simulink model "l1_ver106"  (Simulink 7.9)
settings: Solver=FixedStepDiscrete  SolverMode=SingleTasking  StartTime=0.0  StopTime=2048  RelTol=1e-3
undefined symbols (not defined in the file): sfp
libraries: xbsIndex_r4 x1795, casper_library_downconverter x124, xps_library x32, dspsigops x32, casper_library_misc x23, casper_library_flow_control x16, simulink x7
(part 3 of 16)

== system l1_ver106/converter28 ==
  u8_0 : Inport
  Concat : xbsIndex_r4/Concat  num_inputs=2  dbl_ovrd=off  block_type=concat
  Inverter1 : xbsIndex_r4/Inverter  en=off  latency=0  dbl_ovrd=off  block_type=inv
  Reinterpret : xbsIndex_r4/Reinterpret  force_arith_type=on  arith_type="Signed  (2's comp)"  force_bin_pt=on  bin_pt=7  block_type=reinterpret
  Slice : xbsIndex_r4/Slice  nbits=1  boolean_output=off  mode=Upper Bit Location + Width  bit1=0  base1=MSB of Input  bit0=0  base0=LSB of Input  dbl_ovrd=off  block_type=slice
  Slice1 : xbsIndex_r4/Slice  nbits=7  boolean_output=off  mode=Lower Bit Location + Width  bit1=0  base1=MSB of Input  bit0=0  base0=LSB of Input  dbl_ovrd=off  block_type=slice
  s8_7 : Outport
  wires:
  u8_0/1 -> Slice1/1, Slice/1
  Slice/1 -> Inverter1/1
  Concat/1 -> Reinterpret/1
  Inverter1/1 -> Concat/1
  Slice1/1 -> Concat/2
  Reinterpret/1 -> s8_7/1

== system l1_ver106/converter29 ==
  u8_0 : Inport
  Concat : xbsIndex_r4/Concat  num_inputs=2  dbl_ovrd=off  block_type=concat
  Inverter1 : xbsIndex_r4/Inverter  en=off  latency=0  dbl_ovrd=off  block_type=inv
  Reinterpret : xbsIndex_r4/Reinterpret  force_arith_type=on  arith_type="Signed  (2's comp)"  force_bin_pt=on  bin_pt=7  block_type=reinterpret
  Slice : xbsIndex_r4/Slice  nbits=1  boolean_output=off  mode=Upper Bit Location + Width  bit1=0  base1=MSB of Input  bit0=0  base0=LSB of Input  dbl_ovrd=off  block_type=slice
  Slice1 : xbsIndex_r4/Slice  nbits=7  boolean_output=off  mode=Lower Bit Location + Width  bit1=0  base1=MSB of Input  bit0=0  base0=LSB of Input  dbl_ovrd=off  block_type=slice
  s8_7 : Outport
  wires:
  Reinterpret/1 -> s8_7/1
  Slice1/1 -> Concat/2
  Inverter1/1 -> Concat/1
  Concat/1 -> Reinterpret/1
  Slice/1 -> Inverter1/1
  u8_0/1 -> Slice/1, Slice1/1

== system l1_ver106/converter3 ==
  u8_0 : Inport
  Concat : xbsIndex_r4/Concat  num_inputs=2  dbl_ovrd=off  block_type=concat
  Inverter1 : xbsIndex_r4/Inverter  en=off  latency=0  dbl_ovrd=off  block_type=inv
  Reinterpret : xbsIndex_r4/Reinterpret  force_arith_type=on  arith_type="Signed  (2's comp)"  force_bin_pt=on  bin_pt=7  block_type=reinterpret
  Slice : xbsIndex_r4/Slice  nbits=1  boolean_output=off  mode=Upper Bit Location + Width  bit1=0  base1=MSB of Input  bit0=0  base0=LSB of Input  dbl_ovrd=off  block_type=slice
  Slice1 : xbsIndex_r4/Slice  nbits=7  boolean_output=off  mode=Lower Bit Location + Width  bit1=0  base1=MSB of Input  bit0=0  base0=LSB of Input  dbl_ovrd=off  block_type=slice
  s8_7 : Outport
  wires:
  Reinterpret/1 -> s8_7/1
  Slice1/1 -> Concat/2
  Inverter1/1 -> Concat/1
  Concat/1 -> Reinterpret/1
  Slice/1 -> Inverter1/1
  u8_0/1 -> Slice/1, Slice1/1

== system l1_ver106/converter30 ==
  u8_0 : Inport
  Concat : xbsIndex_r4/Concat  num_inputs=2  dbl_ovrd=off  block_type=concat
  Inverter1 : xbsIndex_r4/Inverter  en=off  latency=0  dbl_ovrd=off  block_type=inv
  Reinterpret : xbsIndex_r4/Reinterpret  force_arith_type=on  arith_type="Signed  (2's comp)"  force_bin_pt=on  bin_pt=7  block_type=reinterpret
  Slice : xbsIndex_r4/Slice  nbits=1  boolean_output=off  mode=Upper Bit Location + Width  bit1=0  base1=MSB of Input  bit0=0  base0=LSB of Input  dbl_ovrd=off  block_type=slice
  Slice1 : xbsIndex_r4/Slice  nbits=7  boolean_output=off  mode=Lower Bit Location + Width  bit1=0  base1=MSB of Input  bit0=0  base0=LSB of Input  dbl_ovrd=off  block_type=slice
  s8_7 : Outport
  wires:
  u8_0/1 -> Slice1/1, Slice/1
  Slice/1 -> Inverter1/1
  Concat/1 -> Reinterpret/1
  Inverter1/1 -> Concat/1
  Slice1/1 -> Concat/2
  Reinterpret/1 -> s8_7/1

== system l1_ver106/converter31 ==
  u8_0 : Inport
  Concat : xbsIndex_r4/Concat  num_inputs=2  dbl_ovrd=off  block_type=concat
  Inverter1 : xbsIndex_r4/Inverter  en=off  latency=0  dbl_ovrd=off  block_type=inv
  Reinterpret : xbsIndex_r4/Reinterpret  force_arith_type=on  arith_type="Signed  (2's comp)"  force_bin_pt=on  bin_pt=7  block_type=reinterpret
  Slice : xbsIndex_r4/Slice  nbits=1  boolean_output=off  mode=Upper Bit Location + Width  bit1=0  base1=MSB of Input  bit0=0  base0=LSB of Input  dbl_ovrd=off  block_type=slice
  Slice1 : xbsIndex_r4/Slice  nbits=7  boolean_output=off  mode=Lower Bit Location + Width  bit1=0  base1=MSB of Input  bit0=0  base0=LSB of Input  dbl_ovrd=off  block_type=slice
  s8_7 : Outport
  wires:
  u8_0/1 -> Slice1/1, Slice/1
  Slice/1 -> Inverter1/1
  Concat/1 -> Reinterpret/1
  Inverter1/1 -> Concat/1
  Slice1/1 -> Concat/2
  Reinterpret/1 -> s8_7/1

== system l1_ver106/converter4 ==
  u8_0 : Inport
  Concat : xbsIndex_r4/Concat  num_inputs=2  dbl_ovrd=off  block_type=concat
  Inverter1 : xbsIndex_r4/Inverter  en=off  latency=0  dbl_ovrd=off  block_type=inv
  Reinterpret : xbsIndex_r4/Reinterpret  force_arith_type=on  arith_type="Signed  (2's comp)"  force_bin_pt=on  bin_pt=7  block_type=reinterpret
  Slice : xbsIndex_r4/Slice  nbits=1  boolean_output=off  mode=Upper Bit Location + Width  bit1=0  base1=MSB of Input  bit0=0  base0=LSB of Input  dbl_ovrd=off  block_type=slice
  Slice1 : xbsIndex_r4/Slice  nbits=7  boolean_output=off  mode=Lower Bit Location + Width  bit1=0  base1=MSB of Input  bit0=0  base0=LSB of Input  dbl_ovrd=off  block_type=slice
  s8_7 : Outport
  wires:
  u8_0/1 -> Slice1/1, Slice/1
  Slice/1 -> Inverter1/1
  Concat/1 -> Reinterpret/1
  Inverter1/1 -> Concat/1
  Slice1/1 -> Concat/2
  Reinterpret/1 -> s8_7/1

== system l1_ver106/converter5 ==
  u8_0 : Inport
  Concat : xbsIndex_r4/Concat  num_inputs=2  dbl_ovrd=off  block_type=concat
  Inverter1 : xbsIndex_r4/Inverter  en=off  latency=0  dbl_ovrd=off  block_type=inv
  Reinterpret : xbsIndex_r4/Reinterpret  force_arith_type=on  arith_type="Signed  (2's comp)"  force_bin_pt=on  bin_pt=7  block_type=reinterpret
  Slice : xbsIndex_r4/Slice  nbits=1  boolean_output=off  mode=Upper Bit Location + Width  bit1=0  base1=MSB of Input  bit0=0  base0=LSB of Input  dbl_ovrd=off  block_type=slice
  Slice1 : xbsIndex_r4/Slice  nbits=7  boolean_output=off  mode=Lower Bit Location + Width  bit1=0  base1=MSB of Input  bit0=0  base0=LSB of Input  dbl_ovrd=off  block_type=slice
  s8_7 : Outport
  wires:
  Reinterpret/1 -> s8_7/1
  Slice1/1 -> Concat/2
  Inverter1/1 -> Concat/1
  Concat/1 -> Reinterpret/1
  Slice/1 -> Inverter1/1
  u8_0/1 -> Slice/1, Slice1/1

== system l1_ver106/converter6 ==
  u8_0 : Inport
  Concat : xbsIndex_r4/Concat  num_inputs=2  dbl_ovrd=off  block_type=concat
  Inverter1 : xbsIndex_r4/Inverter  en=off  latency=0  dbl_ovrd=off  block_type=inv
  Reinterpret : xbsIndex_r4/Reinterpret  force_arith_type=on  arith_type="Signed  (2's comp)"  force_bin_pt=on  bin_pt=7  block_type=reinterpret
  Slice : xbsIndex_r4/Slice  nbits=1  boolean_output=off  mode=Upper Bit Location + Width  bit1=0  base1=MSB of Input  bit0=0  base0=LSB of Input  dbl_ovrd=off  block_type=slice
  Slice1 : xbsIndex_r4/Slice  nbits=7  boolean_output=off  mode=Lower Bit Location + Width  bit1=0  base1=MSB of Input  bit0=0  base0=LSB of Input  dbl_ovrd=off  block_type=slice
  s8_7 : Outport
  wires:
  u8_0/1 -> Slice1/1, Slice/1
  Slice/1 -> Inverter1/1
  Concat/1 -> Reinterpret/1
  Inverter1/1 -> Concat/1
  Slice1/1 -> Concat/2
  Reinterpret/1 -> s8_7/1

== system l1_ver106/converter7 ==
  u8_0 : Inport
  Concat : xbsIndex_r4/Concat  num_inputs=2  dbl_ovrd=off  block_type=concat
  Inverter1 : xbsIndex_r4/Inverter  en=off  latency=0  dbl_ovrd=off  block_type=inv
  Reinterpret : xbsIndex_r4/Reinterpret  force_arith_type=on  arith_type="Signed  (2's comp)"  force_bin_pt=on  bin_pt=7  block_type=reinterpret
  Slice : xbsIndex_r4/Slice  nbits=1  boolean_output=off  mode=Upper Bit Location + Width  bit1=0  base1=MSB of Input  bit0=0  base0=LSB of Input  dbl_ovrd=off  block_type=slice
  Slice1 : xbsIndex_r4/Slice  nbits=7  boolean_output=off  mode=Lower Bit Location + Width  bit1=0  base1=MSB of Input  bit0=0  base0=LSB of Input  dbl_ovrd=off  block_type=slice
  s8_7 : Outport
  wires:
  Reinterpret/1 -> s8_7/1
  Slice1/1 -> Concat/2
  Inverter1/1 -> Concat/1
  Concat/1 -> Reinterpret/1
  Slice/1 -> Inverter1/1
  u8_0/1 -> Slice/1, Slice1/1

== system l1_ver106/converter8 ==
  u8_0 : Inport
  Concat : xbsIndex_r4/Concat  num_inputs=2  dbl_ovrd=off  block_type=concat
  Inverter1 : xbsIndex_r4/Inverter  en=off  latency=0  dbl_ovrd=off  block_type=inv
  Reinterpret : xbsIndex_r4/Reinterpret  force_arith_type=on  arith_type="Signed  (2's comp)"  force_bin_pt=on  bin_pt=7  block_type=reinterpret
  Slice : xbsIndex_r4/Slice  nbits=1  boolean_output=off  mode=Upper Bit Location + Width  bit1=0  base1=MSB of Input  bit0=0  base0=LSB of Input  dbl_ovrd=off  block_type=slice
  Slice1 : xbsIndex_r4/Slice  nbits=7  boolean_output=off  mode=Lower Bit Location + Width  bit1=0  base1=MSB of Input  bit0=0  base0=LSB of Input  dbl_ovrd=off  block_type=slice
  s8_7 : Outport
  wires:
  u8_0/1 -> Slice1/1, Slice/1
  Slice/1 -> Inverter1/1
  Concat/1 -> Reinterpret/1
  Inverter1/1 -> Concat/1
  Slice1/1 -> Concat/2
  Reinterpret/1 -> s8_7/1

== system l1_ver106/converter9 ==
  u8_0 : Inport
  Concat : xbsIndex_r4/Concat  num_inputs=2  dbl_ovrd=off  block_type=concat
  Inverter1 : xbsIndex_r4/Inverter  en=off  latency=0  dbl_ovrd=off  block_type=inv
  Reinterpret : xbsIndex_r4/Reinterpret  force_arith_type=on  arith_type="Signed  (2's comp)"  force_bin_pt=on  bin_pt=7  block_type=reinterpret
  Slice : xbsIndex_r4/Slice  nbits=1  boolean_output=off  mode=Upper Bit Location + Width  bit1=0  base1=MSB of Input  bit0=0  base0=LSB of Input  dbl_ovrd=off  block_type=slice
  Slice1 : xbsIndex_r4/Slice  nbits=7  boolean_output=off  mode=Lower Bit Location + Width  bit1=0  base1=MSB of Input  bit0=0  base0=LSB of Input  dbl_ovrd=off  block_type=slice
  s8_7 : Outport
  wires:
  Reinterpret/1 -> s8_7/1
  Slice1/1 -> Concat/2
  Inverter1/1 -> Concat/1
  Concat/1 -> Reinterpret/1
  Slice/1 -> Inverter1/1
  u8_0/1 -> Slice/1, Slice1/1

== system l1_ver106/dec_fir1 ==
  sync_in : Inport
  real1 : Inport  Port=2
  imag1 : Inport  Port=3
  real2 : Inport  Port=4
  imag2 : Inport  Port=5
  real3 : Inport  Port=6
  imag3 : Inport  Port=7
  real4 : Inport  Port=8
  imag4 : Inport  Port=9
  real5 : Inport  Port=10
  imag5 : Inport  Port=11
  real6 : Inport  Port=12
  imag6 : Inport  Port=13
  real7 : Inport  Port=14
  imag7 : Inport  Port=15
  real8 : Inport  Port=16
  imag8 : Inport  Port=17
  real9 : Inport  Port=18
  imag9 : Inport  Port=19
  real10 : Inport  Port=20
  imag10 : Inport  Port=21
  real11 : Inport  Port=22
  imag11 : Inport  Port=23
  real12 : Inport  Port=24
  imag12 : Inport  Port=25
  real13 : Inport  Port=26
  imag13 : Inport  Port=27
  real14 : Inport  Port=28
  imag14 : Inport  Port=29
  real15 : Inport  Port=30
  imag15 : Inport  Port=31
  real16 : Inport  Port=32
  imag16 : Inport  Port=33
  convert1 : xbsIndex_r4/Convert  gui_display_data_type=Fixed-point  arith_type="Signed  (2's comp)"  n_bits=32  bin_pt=31  float_type=Single  exp_bits=8  fraction_bits=24  quantization="Round  (unbiased: +/- Inf)"  overflow=Wrap  en=off  latency=2  dbl_ovrd=off  pipeline=off  block_type=convert
  convert2 : xbsIndex_r4/Convert  gui_display_data_type=Fixed-point  arith_type="Signed  (2's comp)"  n_bits=32  bin_pt=31  float_type=Single  exp_bits=8  fraction_bits=24  quantization="Round  (unbiased: +/- Inf)"  overflow=Wrap  en=off  latency=2  dbl_ovrd=off  pipeline=off  block_type=convert
  delay : xbsIndex_r4/Delay  rst=off  infoeditControl=Selection of Reset will increase slice count due to use of real FFs and instead of SRLs  en=off  latency=9  dbl_ovrd=off  reg_retiming=off  block_type=delay
  fir_dbl_col1 : Subsystem  AncestorBlock=casper_library_downconverter/fir_dbl_col  mask(fir_dbl_col){n_inputs=16, coeff=[0.23734     0.22808     0.21853     0.20905     0.19939     0.18961     0.17952     0.16949      0.1593     0.14934     0.13944     0.12979     0.12023     0.11094     0.10175     0.53424], add_latency=1, mult_latency=2, coeff_bit_width=25, coeff_bin_pt=24, first_stage_hdl=on, adder_imp=DSP48}
      mask prompts: n_inputs "Inputs"; coeff "Coefficients"; add_latency "Add latency"; mult_latency "Mult Latency"; coeff_bit_width "Coefficient bit width"; coeff_bin_pt "Coefficient binary point"; first_stage_hdl "Implement first stage of adder trees on output as behavioural HDL"; adder_imp "Adder implementation"
      mask init:
      | fir_dbl_col_init(gcb, ...
      |     'n_inputs', n_inputs, ...
      |     'coeff', coeff, ...
      |     'mult_latency', mult_latency, ...
      |     'add_latency', add_latency, ...
      |     'coeff_bit_width', coeff_bit_width, ...
      |     'coeff_bin_pt', coeff_bin_pt,  ...
      |     'first_stage_hdl', first_stage_hdl, ...
      |     'adder_imp', adder_imp);
      mask Help: eval('xlWeb(''http://casper.berkeley.edu/wiki/Fir_dbl_col'')')
      mask StyleString: edit,edit,edit,edit,edit,edit,checkbox,popup(Behavioral|Fabric|DSP48)
  fir_dbl_col2 : Subsystem  AncestorBlock=casper_library_downconverter/fir_dbl_col  mask(fir_dbl_col){n_inputs=16, coeff=[0.32081     0.32018     0.31891     0.31676     0.31411     0.31084     0.30683     0.30225     0.29702     0.29121     0.28491     0.27801     0.27074     0.26309     0.25434     0.24631], add_latency=1, mult_latency=2, coeff_bit_width=25, coeff_bin_pt=24, first_stage_hdl=on, adder_imp=DSP48}
      mask prompts: n_inputs "Inputs"; coeff "Coefficients"; add_latency "Add latency"; mult_latency "Mult Latency"; coeff_bit_width "Coefficient bit width"; coeff_bin_pt "Coefficient binary point"; first_stage_hdl "Implement first stage of adder trees on output as behavioural HDL"; adder_imp "Adder implementation"
      mask init: same as l1_ver106/dec_fir1/fir_dbl_col1
      mask Help: eval('xlWeb(''http://casper.berkeley.edu/wiki/Fir_dbl_col'')')
      mask StyleString: edit,edit,edit,edit,edit,edit,checkbox,popup(Behavioral|Fabric|DSP48)
  imag_sum : Subsystem  AncestorBlock=casper_library_misc/adder_tree  mask(adder_tree){n_inputs=2, latency=1, adder_imp=DSP48, first_stage_hdl=off}
      mask prompts: n_inputs "Number of Inputs"; latency "Add Latency"; adder_imp "Adder implementation"; first_stage_hdl "Use behavioural HDL for first stage of adders"
      mask help:
      | Sums all inputs using a tree of adds and delays.
      |
      | Number of inputs: The number of inputs to the block to be summed
      | Add latency: The number of clock cycles to perform one addition (affects timing)..
      | Adder implementation: choice of fabric or DSP48E core, or behavioral HDL
      | Use behavioural HDL for first stage of adders: Causes the first stage of adders to be absorbed into DSP blocks if multipliers precede them (Virtex 5). Overrides Adder implementation
      mask init:
      | adder_tree_init(gcb, ...
      |     'n_inputs', n_inputs, ...
      |     'latency', latency, ...
      |     'adder_imp', adder_imp, ...
      |     'first_stage_hdl', first_stage_hdl);
      settings: AttributesFormatString=latency 1
      mask Help: eval('xlWeb(''http://casper.berkeley.edu/wiki/Adder_tree'')')
      mask StyleString: edit,edit,popup(Behavioral|Fabric|DSP48),checkbox
  real_sum : Subsystem  AncestorBlock=casper_library_misc/adder_tree  mask(adder_tree){n_inputs=2, latency=1, adder_imp=DSP48, first_stage_hdl=off}
      mask prompts: n_inputs "Number of Inputs"; latency "Add Latency"; adder_imp "Adder implementation"; first_stage_hdl "Use behavioural HDL for first stage of adders"
      mask help:
      | Sums all inputs using a tree of adds and delays.
      |
      | Number of inputs: The number of inputs to the block to be summed
      | Add latency: The number of clock cycles to perform one addition (affects timing)..
      | Adder implementation: choice of fabric or DSP48E core, or behavioral HDL
      | Use behavioural HDL for first stage of adders: Causes the first stage of adders to be absorbed into DSP blocks if multipliers precede them (Virtex 5). Overrides Adder implementation
      mask init:
      | adder_tree_init(gcb, ...
      |     'n_inputs', n_inputs, ...
      |     'latency', latency, ...
      |     'adder_imp', adder_imp, ...
      |     'first_stage_hdl', first_stage_hdl);
      settings: AttributesFormatString=latency 1
      mask Help: eval('xlWeb(''http://casper.berkeley.edu/wiki/Adder_tree'')')
      mask StyleString: edit,edit,popup(Behavioral|Fabric|DSP48),checkbox
  ri_to_c : casper_library_misc/ri_to_c
  shift1 : xbsIndex_r4/Shift  shift_dir=Left  shift_bits=1  en=off  latency=0  precision=Full  arith_type="Signed  (2's comp)"  n_bits=16  bin_pt=14  quantization=Truncate  overflow=Wrap  dbl_ovrd=off  block_type=shift
  shift2 : xbsIndex_r4/Shift  shift_dir=Left  shift_bits=1  en=off  latency=0  precision=Full  arith_type="Signed  (2's comp)"  n_bits=16  bin_pt=14  quantization=Truncate  overflow=Wrap  dbl_ovrd=off  block_type=shift
  sync_out : Outport
  dout : Outport  Port=2
  wires:
  real1/1 -> fir_dbl_col1/1(real1)
  imag1/1 -> fir_dbl_col1/2(imag1)
  real2/1 -> fir_dbl_col1/3(real2)
  imag2/1 -> fir_dbl_col1/4(imag2)
  real3/1 -> fir_dbl_col1/5(real3)
  imag3/1 -> fir_dbl_col1/6(imag3)
  real4/1 -> fir_dbl_col1/7(real4)
  imag4/1 -> fir_dbl_col1/8(imag4)
  real5/1 -> fir_dbl_col1/9(real5)
  imag5/1 -> fir_dbl_col1/10(imag5)
  real6/1 -> fir_dbl_col1/11(real6)
  imag6/1 -> fir_dbl_col1/12(imag6)
  real7/1 -> fir_dbl_col1/13(real7)
  imag7/1 -> fir_dbl_col1/14(imag7)
  real8/1 -> fir_dbl_col1/15(real8)
  imag8/1 -> fir_dbl_col1/16(imag8)
  real9/1 -> fir_dbl_col1/17(real9)
  imag9/1 -> fir_dbl_col1/18(imag9)
  real10/1 -> fir_dbl_col1/19(real10)
  imag10/1 -> fir_dbl_col1/20(imag10)
  real11/1 -> fir_dbl_col1/21(real11)
  imag11/1 -> fir_dbl_col1/22(imag11)
  real12/1 -> fir_dbl_col1/23(real12)
  imag12/1 -> fir_dbl_col1/24(imag12)
  real13/1 -> fir_dbl_col1/25(real13)
  imag13/1 -> fir_dbl_col1/26(imag13)
  real14/1 -> fir_dbl_col1/27(real14)
  imag14/1 -> fir_dbl_col1/28(imag14)
  real15/1 -> fir_dbl_col1/29(real15)
  imag15/1 -> fir_dbl_col1/30(imag15)
  real16/1 -> fir_dbl_col1/31(real16)
  imag16/1 -> fir_dbl_col1/32(imag16)
  fir_dbl_col1/65(real_sum) -> real_sum/2(din1)
  fir_dbl_col1/66(imag_sum) -> imag_sum/2(din1)
  fir_dbl_col1/1(real_out1) -> fir_dbl_col2/1(real1)
  fir_dbl_col1/2(imag_out1) -> fir_dbl_col2/2(imag1)
  fir_dbl_col1/3(real_out2) -> fir_dbl_col2/3(real2)
  fir_dbl_col1/4(imag_out2) -> fir_dbl_col2/4(imag2)
  fir_dbl_col1/5(real_out3) -> fir_dbl_col2/5(real3)
  fir_dbl_col1/6(imag_out3) -> fir_dbl_col2/6(imag3)
  fir_dbl_col1/7(real_out4) -> fir_dbl_col2/7(real4)
  fir_dbl_col1/8(imag_out4) -> fir_dbl_col2/8(imag4)
  fir_dbl_col1/9(real_out5) -> fir_dbl_col2/9(real5)
  fir_dbl_col1/10(imag_out5) -> fir_dbl_col2/10(imag5)
  fir_dbl_col1/11(real_out6) -> fir_dbl_col2/11(real6)
  fir_dbl_col1/12(imag_out6) -> fir_dbl_col2/12(imag6)
  fir_dbl_col1/13(real_out7) -> fir_dbl_col2/13(real7)
  fir_dbl_col1/14(imag_out7) -> fir_dbl_col2/14(imag7)
  fir_dbl_col1/15(real_out8) -> fir_dbl_col2/15(real8)
  fir_dbl_col1/16(imag_out8) -> fir_dbl_col2/16(imag8)
  fir_dbl_col1/17(real_out9) -> fir_dbl_col2/17(real9)
  fir_dbl_col1/18(imag_out9) -> fir_dbl_col2/18(imag9)
  fir_dbl_col1/19(real_out10) -> fir_dbl_col2/19(real10)
  fir_dbl_col1/20(imag_out10) -> fir_dbl_col2/20(imag10)
  fir_dbl_col1/21(real_out11) -> fir_dbl_col2/21(real11)
  fir_dbl_col1/22(imag_out11) -> fir_dbl_col2/22(imag11)
  fir_dbl_col1/23(real_out12) -> fir_dbl_col2/23(real12)
  fir_dbl_col1/24(imag_out12) -> fir_dbl_col2/24(imag12)
  fir_dbl_col1/25(real_out13) -> fir_dbl_col2/25(real13)
  fir_dbl_col1/26(imag_out13) -> fir_dbl_col2/26(imag13)
  fir_dbl_col1/27(real_out14) -> fir_dbl_col2/27(real14)
  fir_dbl_col1/28(imag_out14) -> fir_dbl_col2/28(imag14)
  fir_dbl_col1/29(real_out15) -> fir_dbl_col2/29(real15)
  fir_dbl_col1/30(imag_out15) -> fir_dbl_col2/30(imag15)
  fir_dbl_col1/31(real_out16) -> fir_dbl_col2/31(real16)
  fir_dbl_col1/32(imag_out16) -> fir_dbl_col2/32(imag16)
  fir_dbl_col2/65(real_sum) -> real_sum/3(din2)
  fir_dbl_col2/66(imag_sum) -> imag_sum/3(din2)
  real_sum/2(dout) -> shift1/1
  imag_sum/2(dout) -> shift2/1
  shift1/1 -> convert1/1
  shift2/1 -> convert2/1
  convert1/1 -> ri_to_c/1
  convert2/1 -> ri_to_c/2
  ri_to_c/1 -> dout/1
  sync_in/1 -> delay/1
  delay/1 -> real_sum/1(sync), imag_sum/1(sync)
  real_sum/1(sync_out) -> sync_out/1
  fir_dbl_col2/33(real_back_out1) -> fir_dbl_col1/33(real_back1)
  fir_dbl_col2/34(imag_back_out1) -> fir_dbl_col1/34(imag_back1)
  fir_dbl_col2/35(real_back_out2) -> fir_dbl_col1/35(real_back2)
  fir_dbl_col2/36(imag_back_out2) -> fir_dbl_col1/36(imag_back2)
  fir_dbl_col2/37(real_back_out3) -> fir_dbl_col1/37(real_back3)
  fir_dbl_col2/38(imag_back_out3) -> fir_dbl_col1/38(imag_back3)
  fir_dbl_col2/39(real_back_out4) -> fir_dbl_col1/39(real_back4)
  fir_dbl_col2/40(imag_back_out4) -> fir_dbl_col1/40(imag_back4)
  fir_dbl_col2/41(real_back_out5) -> fir_dbl_col1/41(real_back5)
  fir_dbl_col2/42(imag_back_out5) -> fir_dbl_col1/42(imag_back5)
  fir_dbl_col2/43(real_back_out6) -> fir_dbl_col1/43(real_back6)
  fir_dbl_col2/44(imag_back_out6) -> fir_dbl_col1/44(imag_back6)
  fir_dbl_col2/45(real_back_out7) -> fir_dbl_col1/45(real_back7)
  fir_dbl_col2/46(imag_back_out7) -> fir_dbl_col1/46(imag_back7)
  fir_dbl_col2/47(real_back_out8) -> fir_dbl_col1/47(real_back8)
  fir_dbl_col2/48(imag_back_out8) -> fir_dbl_col1/48(imag_back8)
  fir_dbl_col2/49(real_back_out9) -> fir_dbl_col1/49(real_back9)
  fir_dbl_col2/50(imag_back_out9) -> fir_dbl_col1/50(imag_back9)
  fir_dbl_col2/51(real_back_out10) -> fir_dbl_col1/51(real_back10)
  fir_dbl_col2/52(imag_back_out10) -> fir_dbl_col1/52(imag_back10)
  fir_dbl_col2/53(real_back_out11) -> fir_dbl_col1/53(real_back11)
  fir_dbl_col2/54(imag_back_out11) -> fir_dbl_col1/54(imag_back11)
  fir_dbl_col2/55(real_back_out12) -> fir_dbl_col1/55(real_back12)
  fir_dbl_col2/56(imag_back_out12) -> fir_dbl_col1/56(imag_back12)
  fir_dbl_col2/57(real_back_out13) -> fir_dbl_col1/57(real_back13)
  fir_dbl_col2/58(imag_back_out13) -> fir_dbl_col1/58(imag_back13)
  fir_dbl_col2/59(real_back_out14) -> fir_dbl_col1/59(real_back14)
  fir_dbl_col2/60(imag_back_out14) -> fir_dbl_col1/60(imag_back14)
  fir_dbl_col2/61(real_back_out15) -> fir_dbl_col1/61(real_back15)
  fir_dbl_col2/62(imag_back_out15) -> fir_dbl_col1/62(imag_back15)
  fir_dbl_col2/63(real_back_out16) -> fir_dbl_col1/63(real_back16)
  fir_dbl_col2/64(imag_back_out16) -> fir_dbl_col1/64(imag_back16)
  fir_dbl_col2/1(real_out1) -> fir_dbl_col2/33(real_back1)
  fir_dbl_col2/2(imag_out1) -> fir_dbl_col2/34(imag_back1)
  fir_dbl_col2/3(real_out2) -> fir_dbl_col2/35(real_back2)
  fir_dbl_col2/4(imag_out2) -> fir_dbl_col2/36(imag_back2)
  fir_dbl_col2/5(real_out3) -> fir_dbl_col2/37(real_back3)
  fir_dbl_col2/6(imag_out3) -> fir_dbl_col2/38(imag_back3)
  fir_dbl_col2/7(real_out4) -> fir_dbl_col2/39(real_back4)
  fir_dbl_col2/8(imag_out4) -> fir_dbl_col2/40(imag_back4)
  fir_dbl_col2/9(real_out5) -> fir_dbl_col2/41(real_back5)
  fir_dbl_col2/10(imag_out5) -> fir_dbl_col2/42(imag_back5)
  fir_dbl_col2/11(real_out6) -> fir_dbl_col2/43(real_back6)
  fir_dbl_col2/12(imag_out6) -> fir_dbl_col2/44(imag_back6)
  fir_dbl_col2/13(real_out7) -> fir_dbl_col2/45(real_back7)
  fir_dbl_col2/14(imag_out7) -> fir_dbl_col2/46(imag_back7)
  fir_dbl_col2/15(real_out8) -> fir_dbl_col2/47(real_back8)
  fir_dbl_col2/16(imag_out8) -> fir_dbl_col2/48(imag_back8)
  fir_dbl_col2/17(real_out9) -> fir_dbl_col2/49(real_back9)
  fir_dbl_col2/18(imag_out9) -> fir_dbl_col2/50(imag_back9)
  fir_dbl_col2/19(real_out10) -> fir_dbl_col2/51(real_back10)
  fir_dbl_col2/20(imag_out10) -> fir_dbl_col2/52(imag_back10)
  fir_dbl_col2/21(real_out11) -> fir_dbl_col2/53(real_back11)
  fir_dbl_col2/22(imag_out11) -> fir_dbl_col2/54(imag_back11)
  fir_dbl_col2/23(real_out12) -> fir_dbl_col2/55(real_back12)
  fir_dbl_col2/24(imag_out12) -> fir_dbl_col2/56(imag_back12)
  fir_dbl_col2/25(real_out13) -> fir_dbl_col2/57(real_back13)
  fir_dbl_col2/26(imag_out13) -> fir_dbl_col2/58(imag_back13)
  fir_dbl_col2/27(real_out14) -> fir_dbl_col2/59(real_back14)
  fir_dbl_col2/28(imag_out14) -> fir_dbl_col2/60(imag_back14)
  fir_dbl_col2/29(real_out15) -> fir_dbl_col2/61(real_back15)
  fir_dbl_col2/30(imag_out15) -> fir_dbl_col2/62(imag_back15)
  fir_dbl_col2/31(real_out16) -> fir_dbl_col2/63(real_back16)
  fir_dbl_col2/32(imag_out16) -> fir_dbl_col2/64(imag_back16)

== system l1_ver106/dec_fir3 ==
  sync_in : Inport
  real1 : Inport  Port=2
  imag1 : Inport  Port=3
  real2 : Inport  Port=4
  imag2 : Inport  Port=5
  real3 : Inport  Port=6
  imag3 : Inport  Port=7
  real4 : Inport  Port=8
  imag4 : Inport  Port=9
  real5 : Inport  Port=10
  imag5 : Inport  Port=11
  real6 : Inport  Port=12
  imag6 : Inport  Port=13
  real7 : Inport  Port=14
  imag7 : Inport  Port=15
  real8 : Inport  Port=16
  imag8 : Inport  Port=17
  real9 : Inport  Port=18
  imag9 : Inport  Port=19
  real10 : Inport  Port=20
  imag10 : Inport  Port=21
  real11 : Inport  Port=22
  imag11 : Inport  Port=23
  real12 : Inport  Port=24
  imag12 : Inport  Port=25
  real13 : Inport  Port=26
  imag13 : Inport  Port=27
  real14 : Inport  Port=28
  imag14 : Inport  Port=29
  real15 : Inport  Port=30
  imag15 : Inport  Port=31
  real16 : Inport  Port=32
  imag16 : Inport  Port=33
  convert1 : xbsIndex_r4/Convert  gui_display_data_type=Fixed-point  arith_type="Signed  (2's comp)"  n_bits=32  bin_pt=31  float_type=Single  exp_bits=8  fraction_bits=24  quantization="Round  (unbiased: +/- Inf)"  overflow=Wrap  en=off  latency=2  dbl_ovrd=off  pipeline=off  block_type=convert
  convert2 : xbsIndex_r4/Convert  gui_display_data_type=Fixed-point  arith_type="Signed  (2's comp)"  n_bits=32  bin_pt=31  float_type=Single  exp_bits=8  fraction_bits=24  quantization="Round  (unbiased: +/- Inf)"  overflow=Wrap  en=off  latency=2  dbl_ovrd=off  pipeline=off  block_type=convert
  delay : xbsIndex_r4/Delay  rst=off  infoeditControl=Selection of Reset will increase slice count due to use of real FFs and instead of SRLs  en=off  latency=9  dbl_ovrd=off  reg_retiming=off  block_type=delay
  fir_dbl_col1 : Subsystem  AncestorBlock=casper_library_downconverter/fir_dbl_col  mask(fir_dbl_col){n_inputs=16, coeff=[0.23734     0.22808     0.21853     0.20905     0.19939     0.18961     0.17952     0.16949      0.1593     0.14934     0.13944     0.12979     0.12023     0.11094     0.10175     0.53424], add_latency=1, mult_latency=2, coeff_bit_width=25, coeff_bin_pt=24, first_stage_hdl=on, adder_imp=DSP48}
      mask prompts: n_inputs "Inputs"; coeff "Coefficients"; add_latency "Add latency"; mult_latency "Mult Latency"; coeff_bit_width "Coefficient bit width"; coeff_bin_pt "Coefficient binary point"; first_stage_hdl "Implement first stage of adder trees on output as behavioural HDL"; adder_imp "Adder implementation"
      mask init: same as l1_ver106/dec_fir1/fir_dbl_col1
      mask Help: eval('xlWeb(''http://casper.berkeley.edu/wiki/Fir_dbl_col'')')
      mask StyleString: edit,edit,edit,edit,edit,edit,checkbox,popup(Behavioral|Fabric|DSP48)
  fir_dbl_col2 : Subsystem  AncestorBlock=casper_library_downconverter/fir_dbl_col  mask(fir_dbl_col){n_inputs=16, coeff=[0.32081     0.32018     0.31891     0.31676     0.31411     0.31084     0.30683     0.30225     0.29702     0.29121     0.28491     0.27801     0.27074     0.26309     0.25434     0.24631], add_latency=1, mult_latency=2, coeff_bit_width=25, coeff_bin_pt=24, first_stage_hdl=on, adder_imp=DSP48}
      mask prompts: n_inputs "Inputs"; coeff "Coefficients"; add_latency "Add latency"; mult_latency "Mult Latency"; coeff_bit_width "Coefficient bit width"; coeff_bin_pt "Coefficient binary point"; first_stage_hdl "Implement first stage of adder trees on output as behavioural HDL"; adder_imp "Adder implementation"
      mask init: same as l1_ver106/dec_fir1/fir_dbl_col1
      mask Help: eval('xlWeb(''http://casper.berkeley.edu/wiki/Fir_dbl_col'')')
      mask StyleString: edit,edit,edit,edit,edit,edit,checkbox,popup(Behavioral|Fabric|DSP48)
  imag_sum : Subsystem  AncestorBlock=casper_library_misc/adder_tree  mask(adder_tree){n_inputs=2, latency=1, adder_imp=DSP48, first_stage_hdl=off}
      mask prompts: n_inputs "Number of Inputs"; latency "Add Latency"; adder_imp "Adder implementation"; first_stage_hdl "Use behavioural HDL for first stage of adders"
      mask help:
      | Sums all inputs using a tree of adds and delays.
      |
      | Number of inputs: The number of inputs to the block to be summed
      | Add latency: The number of clock cycles to perform one addition (affects timing)..
      | Adder implementation: choice of fabric or DSP48E core, or behavioral HDL
      | Use behavioural HDL for first stage of adders: Causes the first stage of adders to be absorbed into DSP blocks if multipliers precede them (Virtex 5). Overrides Adder implementation
      mask init:
      | adder_tree_init(gcb, ...
      |     'n_inputs', n_inputs, ...
      |     'latency', latency, ...
      |     'adder_imp', adder_imp, ...
      |     'first_stage_hdl', first_stage_hdl);
      settings: AttributesFormatString=latency 1
      mask Help: eval('xlWeb(''http://casper.berkeley.edu/wiki/Adder_tree'')')
      mask StyleString: edit,edit,popup(Behavioral|Fabric|DSP48),checkbox
  real_sum : Subsystem  AncestorBlock=casper_library_misc/adder_tree  mask(adder_tree){n_inputs=2, latency=1, adder_imp=DSP48, first_stage_hdl=off}
      mask prompts: n_inputs "Number of Inputs"; latency "Add Latency"; adder_imp "Adder implementation"; first_stage_hdl "Use behavioural HDL for first stage of adders"
      mask help:
      | Sums all inputs using a tree of adds and delays.
      |
      | Number of inputs: The number of inputs to the block to be summed
      | Add latency: The number of clock cycles to perform one addition (affects timing)..
      | Adder implementation: choice of fabric or DSP48E core, or behavioral HDL
      | Use behavioural HDL for first stage of adders: Causes the first stage of adders to be absorbed into DSP blocks if multipliers precede them (Virtex 5). Overrides Adder implementation
      mask init:
      | adder_tree_init(gcb, ...
      |     'n_inputs', n_inputs, ...
      |     'latency', latency, ...
      |     'adder_imp', adder_imp, ...
      |     'first_stage_hdl', first_stage_hdl);
      settings: AttributesFormatString=latency 1
      mask Help: eval('xlWeb(''http://casper.berkeley.edu/wiki/Adder_tree'')')
      mask StyleString: edit,edit,popup(Behavioral|Fabric|DSP48),checkbox
  ri_to_c : casper_library_misc/ri_to_c
  shift1 : xbsIndex_r4/Shift  shift_dir=Left  shift_bits=1  en=off  latency=0  precision=Full  arith_type="Signed  (2's comp)"  n_bits=16  bin_pt=14  quantization=Truncate  overflow=Wrap  dbl_ovrd=off  block_type=shift
  shift2 : xbsIndex_r4/Shift  shift_dir=Left  shift_bits=1  en=off  latency=0  precision=Full  arith_type="Signed  (2's comp)"  n_bits=16  bin_pt=14  quantization=Truncate  overflow=Wrap  dbl_ovrd=off  block_type=shift
  sync_out : Outport
  dout : Outport  Port=2
  wires:
  real1/1 -> fir_dbl_col1/1(real1)
  imag1/1 -> fir_dbl_col1/2(imag1)
  real2/1 -> fir_dbl_col1/3(real2)
  imag2/1 -> fir_dbl_col1/4(imag2)
  real3/1 -> fir_dbl_col1/5(real3)
  imag3/1 -> fir_dbl_col1/6(imag3)
  real4/1 -> fir_dbl_col1/7(real4)
  imag4/1 -> fir_dbl_col1/8(imag4)
  real5/1 -> fir_dbl_col1/9(real5)
  imag5/1 -> fir_dbl_col1/10(imag5)
  real6/1 -> fir_dbl_col1/11(real6)
  imag6/1 -> fir_dbl_col1/12(imag6)
  real7/1 -> fir_dbl_col1/13(real7)
  imag7/1 -> fir_dbl_col1/14(imag7)
  real8/1 -> fir_dbl_col1/15(real8)
  imag8/1 -> fir_dbl_col1/16(imag8)
  real9/1 -> fir_dbl_col1/17(real9)
  imag9/1 -> fir_dbl_col1/18(imag9)
  real10/1 -> fir_dbl_col1/19(real10)
  imag10/1 -> fir_dbl_col1/20(imag10)
  real11/1 -> fir_dbl_col1/21(real11)
  imag11/1 -> fir_dbl_col1/22(imag11)
  real12/1 -> fir_dbl_col1/23(real12)
  imag12/1 -> fir_dbl_col1/24(imag12)
  real13/1 -> fir_dbl_col1/25(real13)
  imag13/1 -> fir_dbl_col1/26(imag13)
  real14/1 -> fir_dbl_col1/27(real14)
  imag14/1 -> fir_dbl_col1/28(imag14)
  real15/1 -> fir_dbl_col1/29(real15)
  imag15/1 -> fir_dbl_col1/30(imag15)
  real16/1 -> fir_dbl_col1/31(real16)
  imag16/1 -> fir_dbl_col1/32(imag16)
  fir_dbl_col1/65(real_sum) -> real_sum/2(din1)
  fir_dbl_col1/66(imag_sum) -> imag_sum/2(din1)
  fir_dbl_col1/1(real_out1) -> fir_dbl_col2/1(real1)
  fir_dbl_col1/2(imag_out1) -> fir_dbl_col2/2(imag1)
  fir_dbl_col1/3(real_out2) -> fir_dbl_col2/3(real2)
  fir_dbl_col1/4(imag_out2) -> fir_dbl_col2/4(imag2)
  fir_dbl_col1/5(real_out3) -> fir_dbl_col2/5(real3)
  fir_dbl_col1/6(imag_out3) -> fir_dbl_col2/6(imag3)
  fir_dbl_col1/7(real_out4) -> fir_dbl_col2/7(real4)
  fir_dbl_col1/8(imag_out4) -> fir_dbl_col2/8(imag4)
  fir_dbl_col1/9(real_out5) -> fir_dbl_col2/9(real5)
  fir_dbl_col1/10(imag_out5) -> fir_dbl_col2/10(imag5)
  fir_dbl_col1/11(real_out6) -> fir_dbl_col2/11(real6)
  fir_dbl_col1/12(imag_out6) -> fir_dbl_col2/12(imag6)
  fir_dbl_col1/13(real_out7) -> fir_dbl_col2/13(real7)
  fir_dbl_col1/14(imag_out7) -> fir_dbl_col2/14(imag7)
  fir_dbl_col1/15(real_out8) -> fir_dbl_col2/15(real8)
  fir_dbl_col1/16(imag_out8) -> fir_dbl_col2/16(imag8)
  fir_dbl_col1/17(real_out9) -> fir_dbl_col2/17(real9)
  fir_dbl_col1/18(imag_out9) -> fir_dbl_col2/18(imag9)
  fir_dbl_col1/19(real_out10) -> fir_dbl_col2/19(real10)
  fir_dbl_col1/20(imag_out10) -> fir_dbl_col2/20(imag10)
  fir_dbl_col1/21(real_out11) -> fir_dbl_col2/21(real11)
  fir_dbl_col1/22(imag_out11) -> fir_dbl_col2/22(imag11)
  fir_dbl_col1/23(real_out12) -> fir_dbl_col2/23(real12)
  fir_dbl_col1/24(imag_out12) -> fir_dbl_col2/24(imag12)
  fir_dbl_col1/25(real_out13) -> fir_dbl_col2/25(real13)
  fir_dbl_col1/26(imag_out13) -> fir_dbl_col2/26(imag13)
  fir_dbl_col1/27(real_out14) -> fir_dbl_col2/27(real14)
  fir_dbl_col1/28(imag_out14) -> fir_dbl_col2/28(imag14)
  fir_dbl_col1/29(real_out15) -> fir_dbl_col2/29(real15)
  fir_dbl_col1/30(imag_out15) -> fir_dbl_col2/30(imag15)
  fir_dbl_col1/31(real_out16) -> fir_dbl_col2/31(real16)
  fir_dbl_col1/32(imag_out16) -> fir_dbl_col2/32(imag16)
  fir_dbl_col2/65(real_sum) -> real_sum/3(din2)
  fir_dbl_col2/66(imag_sum) -> imag_sum/3(din2)
  real_sum/2(dout) -> shift1/1
  imag_sum/2(dout) -> shift2/1
  shift1/1 -> convert1/1
  shift2/1 -> convert2/1
  convert1/1 -> ri_to_c/1
  convert2/1 -> ri_to_c/2
  ri_to_c/1 -> dout/1
  sync_in/1 -> delay/1
  delay/1 -> real_sum/1(sync), imag_sum/1(sync)
  real_sum/1(sync_out) -> sync_out/1
  fir_dbl_col2/33(real_back_out1) -> fir_dbl_col1/33(real_back1)
  fir_dbl_col2/34(imag_back_out1) -> fir_dbl_col1/34(imag_back1)
  fir_dbl_col2/35(real_back_out2) -> fir_dbl_col1/35(real_back2)
  fir_dbl_col2/36(imag_back_out2) -> fir_dbl_col1/36(imag_back2)
  fir_dbl_col2/37(real_back_out3) -> fir_dbl_col1/37(real_back3)
  fir_dbl_col2/38(imag_back_out3) -> fir_dbl_col1/38(imag_back3)
  fir_dbl_col2/39(real_back_out4) -> fir_dbl_col1/39(real_back4)
  fir_dbl_col2/40(imag_back_out4) -> fir_dbl_col1/40(imag_back4)
  fir_dbl_col2/41(real_back_out5) -> fir_dbl_col1/41(real_back5)
  fir_dbl_col2/42(imag_back_out5) -> fir_dbl_col1/42(imag_back5)
  fir_dbl_col2/43(real_back_out6) -> fir_dbl_col1/43(real_back6)
  fir_dbl_col2/44(imag_back_out6) -> fir_dbl_col1/44(imag_back6)
  fir_dbl_col2/45(real_back_out7) -> fir_dbl_col1/45(real_back7)
  fir_dbl_col2/46(imag_back_out7) -> fir_dbl_col1/46(imag_back7)
  fir_dbl_col2/47(real_back_out8) -> fir_dbl_col1/47(real_back8)
  fir_dbl_col2/48(imag_back_out8) -> fir_dbl_col1/48(imag_back8)
  fir_dbl_col2/49(real_back_out9) -> fir_dbl_col1/49(real_back9)
  fir_dbl_col2/50(imag_back_out9) -> fir_dbl_col1/50(imag_back9)
  fir_dbl_col2/51(real_back_out10) -> fir_dbl_col1/51(real_back10)
  fir_dbl_col2/52(imag_back_out10) -> fir_dbl_col1/52(imag_back10)
  fir_dbl_col2/53(real_back_out11) -> fir_dbl_col1/53(real_back11)
  fir_dbl_col2/54(imag_back_out11) -> fir_dbl_col1/54(imag_back11)
  fir_dbl_col2/55(real_back_out12) -> fir_dbl_col1/55(real_back12)
  fir_dbl_col2/56(imag_back_out12) -> fir_dbl_col1/56(imag_back12)
  fir_dbl_col2/57(real_back_out13) -> fir_dbl_col1/57(real_back13)
  fir_dbl_col2/58(imag_back_out13) -> fir_dbl_col1/58(imag_back13)
  fir_dbl_col2/59(real_back_out14) -> fir_dbl_col1/59(real_back14)
  fir_dbl_col2/60(imag_back_out14) -> fir_dbl_col1/60(imag_back14)
  fir_dbl_col2/61(real_back_out15) -> fir_dbl_col1/61(real_back15)
  fir_dbl_col2/62(imag_back_out15) -> fir_dbl_col1/62(imag_back15)
  fir_dbl_col2/63(real_back_out16) -> fir_dbl_col1/63(real_back16)
  fir_dbl_col2/64(imag_back_out16) -> fir_dbl_col1/64(imag_back16)
  fir_dbl_col2/1(real_out1) -> fir_dbl_col2/33(real_back1)
  fir_dbl_col2/2(imag_out1) -> fir_dbl_col2/34(imag_back1)
  fir_dbl_col2/3(real_out2) -> fir_dbl_col2/35(real_back2)
  fir_dbl_col2/4(imag_out2) -> fir_dbl_col2/36(imag_back2)
  fir_dbl_col2/5(real_out3) -> fir_dbl_col2/37(real_back3)
  fir_dbl_col2/6(imag_out3) -> fir_dbl_col2/38(imag_back3)
  fir_dbl_col2/7(real_out4) -> fir_dbl_col2/39(real_back4)
  fir_dbl_col2/8(imag_out4) -> fir_dbl_col2/40(imag_back4)
  fir_dbl_col2/9(real_out5) -> fir_dbl_col2/41(real_back5)
  fir_dbl_col2/10(imag_out5) -> fir_dbl_col2/42(imag_back5)
  fir_dbl_col2/11(real_out6) -> fir_dbl_col2/43(real_back6)
  fir_dbl_col2/12(imag_out6) -> fir_dbl_col2/44(imag_back6)
  fir_dbl_col2/13(real_out7) -> fir_dbl_col2/45(real_back7)
  fir_dbl_col2/14(imag_out7) -> fir_dbl_col2/46(imag_back7)
  fir_dbl_col2/15(real_out8) -> fir_dbl_col2/47(real_back8)
  fir_dbl_col2/16(imag_out8) -> fir_dbl_col2/48(imag_back8)
  fir_dbl_col2/17(real_out9) -> fir_dbl_col2/49(real_back9)
  fir_dbl_col2/18(imag_out9) -> fir_dbl_col2/50(imag_back9)
  fir_dbl_col2/19(real_out10) -> fir_dbl_col2/51(real_back10)
  fir_dbl_col2/20(imag_out10) -> fir_dbl_col2/52(imag_back10)
  fir_dbl_col2/21(real_out11) -> fir_dbl_col2/53(real_back11)
  fir_dbl_col2/22(imag_out11) -> fir_dbl_col2/54(imag_back11)
  fir_dbl_col2/23(real_out12) -> fir_dbl_col2/55(real_back12)
  fir_dbl_col2/24(imag_out12) -> fir_dbl_col2/56(imag_back12)
  fir_dbl_col2/25(real_out13) -> fir_dbl_col2/57(real_back13)
  fir_dbl_col2/26(imag_out13) -> fir_dbl_col2/58(imag_back13)
  fir_dbl_col2/27(real_out14) -> fir_dbl_col2/59(real_back14)
  fir_dbl_col2/28(imag_out14) -> fir_dbl_col2/60(imag_back14)
  fir_dbl_col2/29(real_out15) -> fir_dbl_col2/61(real_back15)
  fir_dbl_col2/30(imag_out15) -> fir_dbl_col2/62(imag_back15)
  fir_dbl_col2/31(real_out16) -> fir_dbl_col2/63(real_back16)
  fir_dbl_col2/32(imag_out16) -> fir_dbl_col2/64(imag_back16)

== system l1_ver106/edge_detect5 ==
  in : Inport
  Delay : xbsIndex_r4/Delay  rst=off  infoeditControl=Selection of Reset will increase slice count due to use of real FFs and instead of SRLs  en=off  latency=1  dbl_ovrd=off  reg_retiming=off  block_type=delay
      settings: xl_area=[0, 0, 0, 0, 0, 0, 0]
  Inverter : xbsIndex_r4/Inverter  en=off  latency=0  dbl_ovrd=off  block_type=inv
      settings: xl_area=[0, 0, 0, 0, 0, 0, 0]
  edge_op : xbsIndex_r4/Logical  logical_function=AND  inputs=2  en=off  latency=0  precision=Full  arith_type=Unsigned  n_bits=8  bin_pt=2  align_bp=on  dbl_ovrd=off  block_type=logical
      settings: xl_area=[0, 0, 0, 0, 0, 0, 0]
  out : Outport
  wires:
  Delay/1 -> edge_op/2
  in/1 -> Delay/1, Inverter/1
  edge_op/1 -> out/1
  Inverter/1 -> edge_op/1

== system l1_ver106/filtersnap0 ==
  note: Value on vin
      | aligned with
      | first sample on
      | din captured
  note: Number of valid
      | data samples to
      | skip after trigger
      | before starting
      | capture
  note: Number of
      | bytes in
      | buffer and
      | done bit
  note: Offset in vector
      | from first element
      | captured, used
      | during circular capture
  note: ctrl:
      | bit0: Put a posedge here
      |         to enable a new capture.
      | bit1: ignore external trigger
      |         and trigger immediately.
      | bit2: ignore external write
      |         enable and capture on
      |         every FPGA clock.
      | bit3: continuously capture
      |         data until we get an
      |         external stop command.
  note: Delay start
      | of capture
  note: Generate stop
      | when in circular
      | capture mode
  din : Inport
  we : Inport  Port=2
  trig : Inport  Port=3
  add_del : xbsIndex_r4/Delay  rst=off  infoeditControl=Selection of Reset will increase slice count due to use of real FFs and instead of SRLs  en=off  latency=1  dbl_ovrd=off  reg_retiming=off  block_type=delay
  add_gen : Subsystem  AncestorBlock=casper_library_scopes/snapshot/add_gen  mask{counter_size=12+1+1, increment=2, nsamples=12, burst_size=0, use_dsp48=on}
      mask prompts: counter_size "Address counter size (2^?)"; increment "Address counter increment"; nsamples "Number of samples (2^?)"; burst_size "Burst size (2^?)"; use_dsp48 "dsp48 counter/s"
      mask init:
      | set_param(gcb,'LinkStatus','inactive');
      | if strcmp(use_dsp48,'on'),
      |     set_param([gcb,'/add_gen'],'implementation','DSP48');
      | else
      |     set_param([gcb,'/add_gen'],'implementation','Fabric');
      | end
  basic_ctrl : Subsystem  AncestorBlock=casper_library_scopes/snapshot/basic_ctrl  mask{dram=off, data_width=16}
      mask prompts: data_width "data width"
      mask init:
      | set_param(gcb,'LinkStatus','inactive');
      | set_param([gcb,'/dram_munge'], 'dram', dram);
  bram : Subsystem  Tag=xps:bram  AncestorBlock=xps_library/Shared BRAM  mask{arith_type=Unsigned, addr_width=12, data_width=16, data_bin_pt=0, init_vals=[0:2^10-1], sample_rate=1}
      mask prompts: arith_type "Output Data Type"; addr_width "Address width"; data_width "Data Width"; data_bin_pt "Data Binary Point"; init_vals "Initial 32-bit values (simulation only)"; sample_rate "Sample rate"
      mask init:
      | shared_bram_mask;
      mask StyleString: popup(Unsigned|Signed  (2's comp)),edit,popup(8|16|32|64|128),edit,edit,edit
  cast : xbsIndex_r4/Convert  gui_display_data_type=Fixed-point  arith_type=Unsigned  n_bits=16  bin_pt=0  float_type=Single  exp_bits=8  fraction_bits=24  quantization=Truncate  overflow=Wrap  en=off  latency=0  dbl_ovrd=off  pipeline=off  block_type=convert
  const0 : Constant  Value=0
  ctrl : xps_library/software register  Tag=xps:sw_reg  io_dir=From Processor  arith_type=Unsigned  bitwidth=32  bin_pt=0  sample_period=1  gw_name=l1_ver106_filtersnap0_ctrl_user_data_out
      link override l1_ver101_snapfilter0_ctrl_user_data_out: arith_type=Unsigned  n_bits=32  bin_pt=0  period=1
  dat_del : xbsIndex_r4/Delay  rst=off  infoeditControl=Selection of Reset will increase slice count due to use of real FFs and instead of SRLs  en=off  latency=1  dbl_ovrd=off  reg_retiming=off  block_type=delay
  g_tr_en_cnt : Terminator
  gbram : Terminator
  gstatus : Terminator
  gval : Terminator
  never : xbsIndex_r4/Constant  const=0  gui_display_data_type=Fixed-point  arith_type=Boolean  n_bits=16  bin_pt=14  preci_type=Single  exp_width=8  frac_width=24  explicit_period=on  period=1  equ=P=C  opselect=C  inp2=PCIN>>17  opr=+  inp1=P  carry=CIN  dbl_ovrd=off  block_type=constant
  ri : xbsIndex_r4/Reinterpret  force_arith_type=on  arith_type=Unsigned  force_bin_pt=on  bin_pt=0  block_type=reinterpret
  status : xps_library/software register  Tag=xps:sw_reg  io_dir=To Processor  arith_type=Unsigned  bitwidth=32  bin_pt=0  sample_period=1  gw_name=l1_ver106_filtersnap0_status_user_data_in
      link override convert: arith_type=Unsigned  n_bits=32  bin_pt=0
  vin_const : xbsIndex_r4/Constant  const=0  gui_display_data_type=Fixed-point  arith_type=Boolean  n_bits=16  bin_pt=14  preci_type=Single  exp_width=8  frac_width=24  explicit_period=on  period=1  equ=P=C  opselect=C  inp2=PCIN>>17  opr=+  inp1=P  carry=CIN  dbl_ovrd=off  block_type=constant
  we_del : xbsIndex_r4/Delay  rst=off  infoeditControl=Selection of Reset will increase slice count due to use of real FFs and instead of SRLs  en=off  latency=1  dbl_ovrd=off  reg_retiming=off  block_type=delay
  wires:
  din/1 -> ri/1
  ri/1 -> cast/1
  cast/1 -> basic_ctrl/2(din)
  we/1 -> basic_ctrl/3(we)
  trig/1 -> basic_ctrl/4(trig)
  never/1 -> basic_ctrl/6(stopi)
  const0/1 -> ctrl/1
  ctrl/1 -> basic_ctrl/5(ctrl)
  basic_ctrl/1(vout) -> add_gen/1(vin)
  basic_ctrl/2(dout) -> add_gen/2(din)
  basic_ctrl/3(we_o) -> add_gen/3(we)
  basic_ctrl/4(go) -> add_gen/4(go)
  basic_ctrl/5(stop) -> add_gen/5(cont)
  basic_ctrl/6(init) -> add_gen/6(init)
  add_gen/5(status) -> status/1
  status/1 -> gstatus/1
  vin_const/1 -> basic_ctrl/1(vin)
  add_gen/1(vout) -> gval/1
  add_gen/6(en_cnt) -> g_tr_en_cnt/1
  add_gen/2(add) -> add_del/1
  add_gen/3(dout) -> dat_del/1
  add_gen/4(we_o) -> we_del/1
  add_del/1 -> bram/1(addr)
  dat_del/1 -> bram/2(data_in)
  we_del/1 -> bram/3(we)
  bram/1(data_out) -> gbram/1
